simulink model "TosAeb"  (R2021a)
settings: Solver=FixedStepDiscrete  StartTime=0.0  StopTime=inf  FixedStep=0.01  RelTol=1e-3
undefined symbols (not defined in the file or in its folder): ACCModSts, AccSts1, AEB_ObjectMovingOrientation, AEB_ObjectMovingState, CN_BTCActive, CN_CurrentGearR, CN_CurrentGearR_DHT, CN_PTCActive, CN_VDCActive, CN_VSC_EspOff, CN_VSC_FCW_AEB_Error, CN_VSC_FCW_AEB_NoDispay, CN_VSC_FCW_AEB_PerfmDgdtn, CN_VSC_FCW_AEB_TempUnAvlbl, CN_VSC_VehStandstill, CNaccelAvoidMax, CNaccelAvoidMin, CNbrakePedalPress, CNcomfortStrLeftAccelMax, CNcomfortStrLeftAccelMin, CNcomfortStrRightAccelMax, CNcomfortStrRightAccelMin, CNDrvDoorOpen, CNDrvModeEco, CNDrvModeNorm, CNDrvModeOther, CNDrvModeSport, CNDrvSBRreminder, CNNull, CNobjInvalidID, CNPassDoorOpen, CNPassSBRreminder, CNPEBSAccelNegInf, CNPEBSAccelNull, CNPEBSTimeInf, CNPEBSTimeNull, CNSTOPMAX, CNSTOPNULL, CNsubjWidth, CNSwtOFF, CNSwtON, CNTTCMAX, CNTTCNULL, CNVehConfigAEBCycNone, CNVehConfigAEBNone, CNVehConfigAEBPedNone, CNVehConfigFCWNone, CNVehConfigJANone, CollisionSeverity, FALSE, FCWSnvty, JACycObjCalcInfoDefaultParams, JAPedObjCalcInfoDefaultParams, JAVehObjCalcInfoDefaultParams, latCycObjCalcInfoDefaultParams, latPedObjCalcInfoDefaultParams, longObjCalcInfoDefaultParams, ModSts, movingStatus, ObjectType ... (+1112)
libraries: simulink x3031, AEBSFunctionLib x50, Ta_Library x33, AEBSStateMachineLib x23, Mylibrary x12, simulink_extras x7, ObjSelect_lib x3, dspstat3 x2
(part 8 of 102)

== system TosAeb/FcwAebJa_Step_sys/ALG_AEBSystem/ALG_AEBController/AEB_FuncCondCalcApp/CycFunctionCondCalc ==
  inputSignals : Inport
  Bus Creator : BusCreator  Inputs=8  OutDataTypeStr=Bus: BusCycFuncSuppAborCond
      settings: InheritFromInputs=off
  cycAEBfullSuppAborCond : Subsystem
  cycAEBpartSuppAborCond : Subsystem
  cycPrefillSuppAborCond : Subsystem
  cycFuncSuppAborCond : Outport  OutDataTypeStr=Bus: BusCycFuncSuppAborCond
  wires:
  cycAEBfullSuppAborCond/1(cycAEBfullSuppCond) -> Bus Creator/6  "cycAEBfullSuppCond"
  cycAEBpartSuppAborCond/3(cycAEBpartAborCondStandstill) -> Bus Creator/5  "cycAEBpartAborCondStandstill"
  cycAEBfullSuppAborCond/2(cycAEBfullAborCondNoStandstill) -> Bus Creator/7  "cycAEBfullAborCondNoStandstill"
  cycAEBfullSuppAborCond/3(cycAEBfullAborCondStandstill) -> Bus Creator/8  "cycAEBfullAborCondStandstill"
  cycAEBpartSuppAborCond/2(cycAEBpartAborCondNoStandstill) -> Bus Creator/4  "cycAEBpartAborCondNoStandstill"
  inputSignals/1 -> cycPrefillSuppAborCond/1(inputSignals), cycAEBpartSuppAborCond/1(inputSignals), cycAEBfullSuppAborCond/1(inputSignals)
  cycAEBpartSuppAborCond/1(cycAEBpartSuppCond) -> Bus Creator/3  "cycAEBpartSuppCond"
  cycPrefillSuppAborCond/2(cycPrefillAborCond) -> Bus Creator/2  "cycPrefillAborCond"
  cycPrefillSuppAborCond/1(cycPrefillSuppCond) -> Bus Creator/1  "cycPrefillSuppCond"
  Bus Creator/1 -> cycFuncSuppAborCond/1

== system TosAeb/FcwAebJa_Step_sys/ALG_AEBSystem/ALG_AEBController/AEB_FuncCondCalcApp/JaCycFunctionCondCalc ==
  inputSignals : Inport
  Bus Creator : BusCreator  Inputs=5
  JaCycAEBfullSuppAborCond : Subsystem
  JaCycPrefillSuppAborCond : Subsystem
  JaCycFuncSuppAborCond : Outport
  wires:
  JaCycAEBfullSuppAborCond/3(JaCycAEBfullAborCondStandstill) -> Bus Creator/5  "JACycAEBfullAborCondStandstill"
  inputSignals/1 -> JaCycPrefillSuppAborCond/1(inputSignals), JaCycAEBfullSuppAborCond/1(inputSignals)
  JaCycPrefillSuppAborCond/2(JaCycPrefillAborCond) -> Bus Creator/2  "JACycPrefillAborCond"
  JaCycPrefillSuppAborCond/1(JaCycPrefillSuppCond) -> Bus Creator/1  "JACycPrefillSuppCond"
  Bus Creator/1 -> JaCycFuncSuppAborCond/1
  JaCycAEBfullSuppAborCond/1(JaCycAEBfullSuppCond) -> Bus Creator/3  "JACycAEBfullSuppCond"
  JaCycAEBfullSuppAborCond/2(JaCycAEBfullAborCondNoStandstill) -> Bus Creator/4  "JACycAEBfullAborCondNoStandstill"

== system TosAeb/FcwAebJa_Step_sys/ALG_AEBSystem/ALG_AEBController/AEB_FuncCondCalcApp/JaPedFunctionCondCalc ==
  inputSignals : Inport
  AEBJAPedAEBfullSuppAborCond : Subsystem
  AEBJAPedPrefillSuppAborCond : Subsystem
  Bus Creator : BusCreator  Inputs=5
  AEBJAPedFuncSuppAborCond : Outport
  wires:
  AEBJAPedAEBfullSuppAborCond/2(AEBJAPedAEBfullAborCondNoStandstill) -> Bus Creator/4  "JAPedAEBfullAborCondNoStandstill"
  AEBJAPedAEBfullSuppAborCond/1(AEBJAPedAEBfullSuppCond) -> Bus Creator/3  "JAPedAEBfullSuppCond"
  Bus Creator/1 -> AEBJAPedFuncSuppAborCond/1
  AEBJAPedPrefillSuppAborCond/1(AEBJAPedPrefillSuppCond) -> Bus Creator/1  "JAPedPrefillSuppCond"
  AEBJAPedPrefillSuppAborCond/2(AEBJAPedPrefillAborCond) -> Bus Creator/2  "JAPedPrefillAborCond"
  inputSignals/1 -> AEBJAPedAEBfullSuppAborCond/1(inputSignals), AEBJAPedPrefillSuppAborCond/1(inputSignals)
  AEBJAPedAEBfullSuppAborCond/3(AEBJAPedAEBfullAborCondStandstill) -> Bus Creator/5  "JAPedAEBfullAborCondStandstill"

== system TosAeb/FcwAebJa_Step_sys/ALG_AEBSystem/ALG_AEBController/AEB_FuncCondCalcApp/JaVehFunctionCondCalc ==
  inputSignals : Inport
  AEBJAVehAEBfullSuppAborCond : Subsystem
  AEBJAVehPrefillSuppAborCond : Subsystem
  Bus Creator : BusCreator  Inputs=5
  AEBJAVehFuncSuppAborCond : Outport
  wires:
  AEBJAVehAEBfullSuppAborCond/3(AEBJAVehAEBfullAborCondStandstill) -> Bus Creator/5  "JAVehAEBfullAborCondStandstill"
  inputSignals/1 -> AEBJAVehPrefillSuppAborCond/1(inputSignals), AEBJAVehAEBfullSuppAborCond/1(inputSignals)
  AEBJAVehPrefillSuppAborCond/2(AEBJAVehPrefillAborCond) -> Bus Creator/2  "JAVehPrefillAborCond"
  AEBJAVehPrefillSuppAborCond/1(AEBJAVehPrefillSuppCond) -> Bus Creator/1  "JAVehPrefillSuppCond"
  Bus Creator/1 -> AEBJAVehFuncSuppAborCond/1
  AEBJAVehAEBfullSuppAborCond/1(AEBJAVehAEBfullSuppCond) -> Bus Creator/3  "JAVehAEBfullSuppCond"
  AEBJAVehAEBfullSuppAborCond/2(AEBJAVehAEBfullAborCondNoStandstill) -> Bus Creator/4  "JAVehAEBfullAborCondNoStandstill"

== system TosAeb/FcwAebJa_Step_sys/ALG_AEBSystem/ALG_AEBController/AEB_FuncCondCalcApp/LongFunctionCondCalc ==
  inputSignals : Inport
  Bus Creator1 : BusCreator  Inputs=19  OutDataTypeStr=Bus: BusVehFuncSuppAborCond
      settings: InheritFromInputs=off
  vehABASuppAborCondCalc : Subsystem
  vehAEBfullSuppAborCondCalc : Subsystem
  vehAEBpartSuppAborCondCalc : Subsystem
  vehAcuteWarningSuppAborCondFulfilled : Subsystem
  vehEBASuppAborCondCalc : Subsystem
  vehLatentWarningSuppAborCondFulfilled : Subsystem
  vehPreWarningSuppAborCondFulfilled : Subsystem
  vehPrefillSuppAborCondCalc : Subsystem
  vehFuncSuppAborCond : Outport  OutDataTypeStr=Bus: BusVehFuncSuppAborCond
  wires:
  Bus Creator1/1 -> vehFuncSuppAborCond/1  "vehFuncSuppAborCond"
  vehAEBpartSuppAborCondCalc/3(vehAEBpartAborCondStandstill) -> Bus Creator1/13  "vehAEBpartAborCondStandstill"
  vehAEBpartSuppAborCondCalc/2(vehAEBpartAborCondNoStandstill) -> Bus Creator1/12  "vehAEBpartAborCondNoStandstill"
  vehPrefillSuppAborCondCalc/2(vehPrefillAborCond) -> Bus Creator1/8  "vehPrefillAborCond"
  vehAEBpartSuppAborCondCalc/1(vehAEBpartSuppCond) -> Bus Creator1/11  "vehAEBpartSuppCond"
  vehABASuppAborCondCalc/1(vehABASuppCond) -> Bus Creator1/9  "vehABASuppCond"
  vehAEBfullSuppAborCondCalc/2(vehAEBfullAborCondNoStandstill) -> Bus Creator1/15  "vehAEBfullAborCondNoStandstill"
  vehEBASuppAborCondCalc/1(vehEBASuppCond) -> Bus Creator1/17  "vehEBASuppCond"
  vehAEBfullSuppAborCondCalc/3(vehAEBfullAborCondStandstill) -> Bus Creator1/16  "vehAEBfullAborCondStandstill"
  vehPrefillSuppAborCondCalc/1(vehPrefillSuppCond) -> Bus Creator1/7  "vehPrefillSuppAborCond"
  vehAEBfullSuppAborCondCalc/1(vehAEBfullSuppCond) -> Bus Creator1/14  "vehAEBfullSuppCond"
  vehABASuppAborCondCalc/2(vehABAAborCond) -> Bus Creator1/10  "vehABAAborCond"
  vehEBASuppAborCondCalc/2(vehEBAAborCondNoStandstill) -> Bus Creator1/18  "vehEBAAborCondNoStandstill"
  vehEBASuppAborCondCalc/3(vehEBAAborCondStandstill) -> Bus Creator1/19  "vehEBAAborCondStandstill"
  vehLatentWarningSuppAborCondFulfilled/2(vehLatentWarningAborCond) -> Bus Creator1/6  "vehLatentWarningAborCond"
  vehLatentWarningSuppAborCondFulfilled/1(vehLatentWarningSuppCond) -> Bus Creator1/5  "vehLatentWarningSuppCond"
  vehAcuteWarningSuppAborCondFulfilled/2(vehAcuteWarningAborCond) -> Bus Creator1/4  "vehAcuteWarningAborCond"
  vehAcuteWarningSuppAborCondFulfilled/1(vehAcuteWarningSuppCond) -> Bus Creator1/3  "vehAcuteWarningSuppCond"
  vehPreWarningSuppAborCondFulfilled/2(vehPreWarningAborCond) -> Bus Creator1/2  "vehPreWarningAborCond"
  vehPreWarningSuppAborCondFulfilled/1(vehPreWarningSuppCond) -> Bus Creator1/1  "vehPreWarningSuppCond"
  inputSignals/1 -> vehPreWarningSuppAborCondFulfilled/1(inputSignals), vehAcuteWarningSuppAborCondFulfilled/1(inputSignals), vehLatentWarningSuppAborCondFulfilled/1(inputSignals), vehPrefillSuppAborCondCalc/1(inputSignals), vehABASuppAborCondCalc/1(inputSignals), vehAEBpartSuppAborCondCalc/1(inputSignals), vehAEBfullSuppAborCondCalc/1(inputSignals), vehEBASuppAborCondCalc/1(inputSignals)

== system TosAeb/FcwAebJa_Step_sys/ALG_AEBSystem/ALG_AEBController/AEB_FuncCondCalcApp/PedFunctionCondCalc ==
  inputSignals : Inport
  Bus Creator : BusCreator  Inputs=9  OutDataTypeStr=Bus: BusPedFuncSuppAborCond
      settings: InheritFromInputs=off
  pedABASuppAborCond : Subsystem
  pedAEBfullSuppAborCond : Subsystem
  pedPreWarningSuppAborCond : Subsystem
  pedPrefillSuppAborCond : Subsystem
  pedFuncSuppAborCond : Outport  OutDataTypeStr=Bus: BusPedFuncSuppAborCond
  wires:
  pedAEBfullSuppAborCond/3(pedAEBfullAborCondStandstill) -> Bus Creator/9  "pedAEBfullAborCondStandstill"
  pedAEBfullSuppAborCond/2(pedAEBfullAborCondNoStandstill) -> Bus Creator/8  "pedAEBfullAborCondNoStandstill"
  pedAEBfullSuppAborCond/1(pedAEBfullSuppCond) -> Bus Creator/7  "pedAEBfullSuppCond"
  pedABASuppAborCond/2(pedABAAborCond) -> Bus Creator/6  "pedABAAborCond"
  pedABASuppAborCond/1(pedABASuppCond) -> Bus Creator/5  "pedABASuppCond"
  Bus Creator/1 -> pedFuncSuppAborCond/1
  pedPrefillSuppAborCond/1(pedPrefillSuppCond) -> Bus Creator/1  "pedPrefillSuppCond"
  pedPrefillSuppAborCond/2(pedPrefillAborCond) -> Bus Creator/2  "pedPrefillAborCond"
  pedPreWarningSuppAborCond/2(pedPreWarningAborCond) -> Bus Creator/4  "pedPreWarningAborCond"
  pedPreWarningSuppAborCond/1(pedPreWarningSuppCond) -> Bus Creator/3  "pedPreWarningSuppCond"
  inputSignals/1 -> pedAEBfullSuppAborCond/1(inputSignals), pedABASuppAborCond/1(inputSignals), pedPreWarningSuppAborCond/1(inputSignals), pedPrefillSuppAborCond/1(inputSignals)

== system TosAeb/FcwAebJa_Step_sys/ALG_AEBSystem/ALG_AEBController/AEB_FuncCondCalcInfo_FUS/CycFunctionCondCalc ==
  inputSignals : Inport
  Bus Creator : BusCreator  Inputs=8
  cycAEBfullSuppAborCond : Subsystem
  cycAEBpartSuppAborCond : Subsystem
  cycPrefillSuppAborCond : Subsystem
  cycFuncSuppAborCond : Outport
  wires:
  cycAEBfullSuppAborCond/1(cycAEBfullSuppCond) -> Bus Creator/6  "cycAEBfullSuppCond"
  cycAEBpartSuppAborCond/3(cycAEBpartAborCondStandstill) -> Bus Creator/5  "cycAEBpartAborCondStandstill"
  cycAEBfullSuppAborCond/2(cycAEBfullAborCondNoStandstill) -> Bus Creator/7  "cycAEBfullAborCondNoStandstill"
  cycAEBfullSuppAborCond/3(cycAEBfullAborCondStandstill) -> Bus Creator/8  "cycAEBfullAborCondStandstill"
  cycAEBpartSuppAborCond/2(cycAEBpartAborCondNoStandstill) -> Bus Creator/4  "cycAEBpartAborCondNoStandstill"
  inputSignals/1 -> cycPrefillSuppAborCond/1(inputSignals), cycAEBpartSuppAborCond/1(inputSignals), cycAEBfullSuppAborCond/1(inputSignals)
  cycAEBpartSuppAborCond/1(cycAEBpartSuppCond) -> Bus Creator/3  "cycAEBpartSuppCond"
  cycPrefillSuppAborCond/2(cycPrefillAborCond) -> Bus Creator/2  "cycPrefillAborCond"
  cycPrefillSuppAborCond/1(cycPrefillSuppCond) -> Bus Creator/1  "cycPrefillSuppCond"
  Bus Creator/1 -> cycFuncSuppAborCond/1

== system TosAeb/FcwAebJa_Step_sys/ALG_AEBSystem/ALG_AEBController/AEB_FuncCondCalcInfo_FUS/JaCycFunctionCondCalc ==
  inputSignals : Inport
  Bus Creator : BusCreator  Inputs=5
  JaCycAEBfullSuppAborCond : Subsystem
  JaCycPrefillSuppAborCond : Subsystem
  JaCycFuncSuppAborCond : Outport
  wires:
  JaCycAEBfullSuppAborCond/3(JaCycAEBfullAborCondStandstill) -> Bus Creator/5  "JACycAEBfullAborCondStandstill"
  inputSignals/1 -> JaCycPrefillSuppAborCond/1(inputSignals), JaCycAEBfullSuppAborCond/1(inputSignals)
  JaCycPrefillSuppAborCond/2(JaCycPrefillAborCond) -> Bus Creator/2  "JACycPrefillAborCond"
  JaCycPrefillSuppAborCond/1(JaCycPrefillSuppCond) -> Bus Creator/1  "JACycPrefillSuppCond"
  Bus Creator/1 -> JaCycFuncSuppAborCond/1
  JaCycAEBfullSuppAborCond/1(JaCycAEBfullSuppCond) -> Bus Creator/3  "JACycAEBfullSuppCond"
  JaCycAEBfullSuppAborCond/2(JaCycAEBfullAborCondNoStandstill) -> Bus Creator/4  "JACycAEBfullAborCondNoStandstill"

== system TosAeb/FcwAebJa_Step_sys/ALG_AEBSystem/ALG_AEBController/AEB_FuncCondCalcInfo_FUS/JaPedFunctionCondCalc ==
  inputSignals : Inport
  AEBJAPedAEBfullSuppAborCond : Subsystem
  AEBJAPedPrefillSuppAborCond : Subsystem
  Bus Creator : BusCreator  Inputs=5
  AEBJAPedFuncSuppAborCond : Outport
  wires:
  AEBJAPedAEBfullSuppAborCond/2(AEBJAPedAEBfullAborCondNoStandstill) -> Bus Creator/4  "JAPedAEBfullAborCondNoStandstill"
  AEBJAPedAEBfullSuppAborCond/1(AEBJAPedAEBfullSuppCond) -> Bus Creator/3  "JAPedAEBfullSuppCond"
  Bus Creator/1 -> AEBJAPedFuncSuppAborCond/1
  AEBJAPedPrefillSuppAborCond/1(JaPedPrefillSuppCond) -> Bus Creator/1  "JAPedPrefillSuppCond"
  AEBJAPedPrefillSuppAborCond/2(JaPedPrefillAborCond) -> Bus Creator/2  "JAPedPrefillAborCond"
  inputSignals/1 -> AEBJAPedAEBfullSuppAborCond/1(inputSignals), AEBJAPedPrefillSuppAborCond/1(inputSignals)
  AEBJAPedAEBfullSuppAborCond/3(AEBJAPedAEBfullAborCondStandstill) -> Bus Creator/5  "JAPedAEBfullAborCondStandstill"

== system TosAeb/FcwAebJa_Step_sys/ALG_AEBSystem/ALG_AEBController/AEB_FuncCondCalcInfo_FUS/JaVehFunctionCondCalc ==
  inputSignals : Inport
  Bus Creator : BusCreator  Inputs=5
  JaVehAEBfullSuppAborCond : Subsystem
  JaVehPrefillSuppAborCond : Subsystem
  AEBJAVehFuncSuppAborCond : Outport
  wires:
  JaVehAEBfullSuppAborCond/3(JaVehAEBfullAborCondStandstill) -> Bus Creator/5  "JAVehAEBfullAborCondStandstill"
  inputSignals/1 -> JaVehPrefillSuppAborCond/1(inputSignals), JaVehAEBfullSuppAborCond/1(inputSignals)
  JaVehPrefillSuppAborCond/2(JaVehPrefillAborCond) -> Bus Creator/2  "JAVehPrefillAborCond"
  JaVehPrefillSuppAborCond/1(JaVehPrefillSuppCond) -> Bus Creator/1  "JAVehPrefillSuppCond"
  Bus Creator/1 -> AEBJAVehFuncSuppAborCond/1
  JaVehAEBfullSuppAborCond/1(JaVehAEBfullSuppCond) -> Bus Creator/3  "JAVehAEBfullSuppCond"
  JaVehAEBfullSuppAborCond/2(JaVehAEBfullAborCondNoStandstill) -> Bus Creator/4  "JAVehAEBfullAborCondNoStandstill"

== system TosAeb/FcwAebJa_Step_sys/ALG_AEBSystem/ALG_AEBController/AEB_FuncCondCalcInfo_FUS/LongFunctionCondCalc ==
  inputSignals : Inport
  Bus Creator1 : BusCreator  Inputs=19  OutDataTypeStr=Bus: BusVehFuncSuppAborCond
      settings: InheritFromInputs=off
  vehABASuppAborCondCalc : Subsystem
  vehAEBfullSuppAborCondCalc : Subsystem
  vehAEBpartSuppAborCondCalc : Subsystem
  vehAcuteWarningSuppAborCondFulfilled : Subsystem
  vehEBASuppAborCondCalc : Subsystem
  vehLatentWarningSuppAborCondFulfilled : Subsystem
  vehPreWarningSuppAborCondFulfilled : Subsystem
  vehPrefillSuppAborCondCalc : Subsystem
  vehFuncSuppAborCond : Outport
  wires:
  Bus Creator1/1 -> vehFuncSuppAborCond/1  "vehFuncSuppAborCond"
  vehAEBpartSuppAborCondCalc/3(vehAEBpartAborCondStandstill) -> Bus Creator1/13  "vehAEBpartAborCondStandstill"
  vehAEBpartSuppAborCondCalc/2(vehAEBpartAborCondNoStandstill) -> Bus Creator1/12  "vehAEBpartAborCondNoStandstill"
  vehPrefillSuppAborCondCalc/2(vehPrefillAborCond) -> Bus Creator1/8  "vehPrefillAborCond"
  vehAEBpartSuppAborCondCalc/1(vehAEBpartSuppCond) -> Bus Creator1/11  "vehAEBpartSuppCond"
  vehABASuppAborCondCalc/1(vehABASuppCond) -> Bus Creator1/9  "vehABASuppCond"
  vehAEBfullSuppAborCondCalc/2(vehAEBfullAborCondNoStandstill) -> Bus Creator1/15  "vehAEBfullAborCondNoStandstill"
  vehEBASuppAborCondCalc/1(vehEBASuppCond) -> Bus Creator1/17  "vehEBASuppCond"
  vehAEBfullSuppAborCondCalc/3(vehAEBfullAborCondStandstill) -> Bus Creator1/16  "vehAEBfullAborCondStandstill"
  vehPrefillSuppAborCondCalc/1(vehPrefillSuppCond) -> Bus Creator1/7  "vehPrefillSuppAborCond"
  vehAEBfullSuppAborCondCalc/1(vehAEBfullSuppCond) -> Bus Creator1/14  "vehAEBfullSuppCond"
  vehABASuppAborCondCalc/2(vehABAAborCond) -> Bus Creator1/10  "vehABAAborCond"
  vehEBASuppAborCondCalc/2(vehEBAAborCondNoStandstill) -> Bus Creator1/18  "vehEBAAborCondNoStandstill"
  vehEBASuppAborCondCalc/3(vehEBAAborCondStandstill) -> Bus Creator1/19  "vehEBAAborCondStandstill"
  vehLatentWarningSuppAborCondFulfilled/2(vehLatentWarningAborCond) -> Bus Creator1/6  "vehLatentWarningAborCond"
  vehLatentWarningSuppAborCondFulfilled/1(vehLatentWarningSuppCond) -> Bus Creator1/5  "vehLatentWarningSuppCond"
  vehAcuteWarningSuppAborCondFulfilled/2(vehAcuteWarningAborCond) -> Bus Creator1/4  "vehAcuteWarningAborCond"
  vehAcuteWarningSuppAborCondFulfilled/1(vehAcuteWarningSuppCond) -> Bus Creator1/3  "vehAcuteWarningSuppCond"
  vehPreWarningSuppAborCondFulfilled/2(vehPreWarningAborCond) -> Bus Creator1/2  "vehPreWarningAborCond"
  vehPreWarningSuppAborCondFulfilled/1(vehPreWarningSuppCond) -> Bus Creator1/1  "vehPreWarningSuppCond"
  inputSignals/1 -> vehPreWarningSuppAborCondFulfilled/1(inputSignals), vehLatentWarningSuppAborCondFulfilled/1(inputSignals), vehPrefillSuppAborCondCalc/1(inputSignals), vehABASuppAborCondCalc/1(inputSignals), vehAEBpartSuppAborCondCalc/1(inputSignals), vehAEBfullSuppAborCondCalc/1(inputSignals), vehEBASuppAborCondCalc/1(inputSignals), vehAcuteWarningSuppAborCondFulfilled/1(inputSignals)

== system TosAeb/FcwAebJa_Step_sys/ALG_AEBSystem/ALG_AEBController/AEB_FuncCondCalcInfo_FUS/PedFunctionCondCalc ==
  inputSignals : Inport
  Bus Creator : BusCreator  Inputs=9
  pedABASuppAborCond : Subsystem
  pedAEBfullSuppAborCond : Subsystem
  pedPreWarningSuppAborCond : Subsystem
  pedPrefillSuppAborCond : Subsystem
  pedFuncSuppAborCond : Outport
  wires:
  pedAEBfullSuppAborCond/3(pedAEBfullAborCondStandstill) -> Bus Creator/9  "pedAEBfullAborCondStandstill"
  pedAEBfullSuppAborCond/2(pedAEBfullAborCondNoStandstill) -> Bus Creator/8  "pedAEBfullAborCondNoStandstill"
  pedAEBfullSuppAborCond/1(pedAEBfullSuppCond) -> Bus Creator/7  "pedAEBfullSuppCond"
  pedABASuppAborCond/2(pedABAAborCond) -> Bus Creator/6  "pedABAAborCond"
  pedABASuppAborCond/1(pedABASuppCond) -> Bus Creator/5  "pedABASuppCond"
  Bus Creator/1 -> pedFuncSuppAborCond/1
  pedPrefillSuppAborCond/1(pedPrefillSuppCond) -> Bus Creator/1  "pedPrefillSuppCond"
  pedPrefillSuppAborCond/2(pedPrefillAborCond) -> Bus Creator/2  "pedPrefillAborCond"
  pedPreWarningSuppAborCond/2(pedPreWarningAborCond) -> Bus Creator/4  "pedPreWarningAborCond"
  pedPreWarningSuppAborCond/1(pedPreWarningSuppCond) -> Bus Creator/3  "pedPreWarningSuppCond"
  inputSignals/1 -> pedAEBfullSuppAborCond/1(inputSignals), pedABASuppAborCond/1(inputSignals), pedPreWarningSuppAborCond/1(inputSignals), pedPrefillSuppAborCond/1(inputSignals)

== system TosAeb/FcwAebJa_Step_sys/ALG_AEBSystem/ALG_AEBController/AEB_FuncImpManagementApp/FuncBlockTimeCalc ==
  AEBModStsFuncImpArb : Inport  OutDataTypeStr=Bus: BusAEBModStsFuncImpArb
  Bus Creator : BusCreator  Inputs=3  OutDataTypeStr=Bus: BusFuncBlockTime  NonVirtualBus=on
      settings: InheritFromInputs=off
  tBlockTimeBrkCalc : Subsystem
  tBlockTimeBrkReadyCalc : Subsystem
  tBlockTimeWarningCalc : Subsystem
  funcBlockTime : Outport  OutDataTypeStr=Bus: BusFuncBlockTime
  wires:
  Bus Creator/1 -> funcBlockTime/1
  tBlockTimeBrkCalc/1(tBlockTimeBrk) -> Bus Creator/3  "tBlockTimeBrk"
  tBlockTimeWarningCalc/1(tBlockTimeWarning) -> Bus Creator/2  "tBlockTimeWarning"
  tBlockTimeBrkReadyCalc/1(tBlockTimeBrkReady) -> Bus Creator/1  "tBlockTimeBrkReady"
  AEBModStsFuncImpArb/1 -> tBlockTimeBrkReadyCalc/1(AEBModStsFuncImpArb), tBlockTimeWarningCalc/1(AEBModStsFuncImpArb), tBlockTimeBrkCalc/1(AEBModStsFuncImpArb)

== system TosAeb/FcwAebJa_Step_sys/ALG_AEBSystem/ALG_AEBController/AEB_FuncImpManagementApp/FuncImpArbCalc ==
  AEBModStsArb : Inport  OutDataTypeStr=Bus: BusAEBModStsArb
  FCTBModStsArb : Inport  Port=2  OutDataTypeStr=Enum: ModSts
  FCTB_ABAModStsArb : Inport  Port=3  OutDataTypeStr=Enum: ModSts
  FCTB_PrefillModStsArb : Inport  Port=4  OutDataTypeStr=Enum: ModSts
  BrkFuncImpArbCalc : Subsystem
  BrkFuncImpArbCalc1 : Subsystem  Commented=on
  BrkReadyFuncImpArbCalc : Subsystem
  BrkReadyFuncImpArbCalc1 : Subsystem  Commented=on
  Bus Creator : BusCreator  Inputs=3  OutDataTypeStr=Bus: BusAEBModStsFuncImpArb  NonVirtualBus=on
      settings: InheritFromInputs=off
  Bus Creator1 : BusCreator  Inputs=3  OutDataTypeStr=Bus: BusFuncImpSuppAborCond  NonVirtualBus=on
      settings: InheritFromInputs=off
  Bus Selector : BusSelector  OutputSignals=vehAEBModStsArb.prefillModStsArb,vehAEBModStsArb.ABAModStsArb,latAEBModStsArb.pedPrefillModStsArb,latAEBModStsArb.pedABAModStsArb,latAEBModStsArb.cycPrefillModStsArb,JA_AEBModStsArb.JA_vehPrefillModStsArb,JA_AEBModStsArb.JA_PTW_PrefillModStsArb,JA_AEBModStsArb.JA_cycPrefillModStsArb,JA_AEBModStsArb.JA_pedPrefillModStsArb
  Bus Selector1 : BusSelector  OutputSignals=vehAEBModStsArb.AEBpartModStsArb,vehAEBModStsArb.AEBfullModStsArb,vehAEBModStsArb.EBAModStsArb,latAEBModStsArb.cycAEBpartModStsArb,latAEBModStsArb.cycAEBfullModStsArb,latAEBModStsArb.pedAEBfullModStsArb,JA_AEBModStsArb.JA_vehAEBModStsArb,JA_AEBModStsArb.JA_PTW_AEBModStsArb,JA_AEBModStsArb.JA_cycAEBModStsArb,JA_AEBModStsArb.JA_pedAEBModStsArb,JA_AEBModStsArb.JA_pedAEBModStsArb,JA_AEBModStsArb.JA_cycAEBModStsArb,JA_AEBModStsArb.JA_PTW_AEBModStsArb
  Terminator : Terminator
  WarningFuncImpArbCalc : Subsystem
  AEBModStsFuncImpArb : Outport
  FuncImpSuppCondAbor : Outport  Port=2
  wires:
  FCTBModStsArb/1 -> BrkFuncImpArbCalc/14(FCTBModStsArb)
  Bus Selector1/13 -> BrkFuncImpArbCalc/13(JA_PTW_AEBfullModStsArb)  "<JA_PTW_AEBModStsArb>"
  Bus Selector1/12 -> BrkFuncImpArbCalc/12(JA_cycAEBfullModStsArb)  "<JA_cycAEBModStsArb>"
  Bus Selector1/11 -> BrkFuncImpArbCalc/11(JA_pedAEBfullModStsArb)  "<JA_pedAEBModStsArb>"
  Bus Selector1/10 -> BrkFuncImpArbCalc/10(JA_pedAEBModStsArb)  "<JA_pedAEBModStsArb>"
  Bus Selector1/9 -> BrkFuncImpArbCalc/9(JA_cycAEBModStsArb)  "<JA_cycAEBModStsArb>"
  Bus Selector1/8 -> BrkFuncImpArbCalc/8(JA_PTW_AEBModStsArb)  "<JA_PTW_AEBModStsArb>"
  Bus Selector1/7 -> BrkFuncImpArbCalc/7(JA_vehAEBModStsArb)  "<JA_vehAEBModStsArb>"
  Bus Selector1/6 -> BrkFuncImpArbCalc/6(pedAEBfullModStsArb)  "<pedAEBfullModStsArb>"
  Bus Selector1/5 -> BrkFuncImpArbCalc/5(cycAEBfullModStsArb)  "<cycAEBfullModStsArb>"
  Bus Selector1/4 -> BrkFuncImpArbCalc/4(cycAEBpartModStsArb)  "<cycAEBpartModStsArb>"
  Bus Selector1/3 -> BrkFuncImpArbCalc/3(EBAModStsArb)  "<EBAModStsArb>"
  Bus Selector1/2 -> BrkFuncImpArbCalc/2(AEBfullModStsArb)  "<AEBfullModStsArb>"
  Bus Selector1/1 -> BrkFuncImpArbCalc/1(AEBpartModStsArb)  "<AEBpartModStsArb>"
  FCTB_PrefillModStsArb/1 -> BrkReadyFuncImpArbCalc/10(FCTB_PrefillModStsArb)  "FCTB_PrefillModStsArb"
  FCTB_ABAModStsArb/1 -> BrkReadyFuncImpArbCalc/11(FCTB_ABAModStsArb)  "FCTB_ABAModStsArb"
  Bus Selector/9 -> BrkReadyFuncImpArbCalc/9(JA_pedPrefillModStsArb)  "<JA_pedPrefillModStsArb>"
  Bus Selector/8 -> BrkReadyFuncImpArbCalc/8(JA_cycPrefillModStsArb)  "<JA_cycPrefillModStsArb>"
  Bus Selector/7 -> BrkReadyFuncImpArbCalc/7(JA_PTW_PrefillModStsArb)  "<JA_PTW_PrefillModStsArb>"
  Bus Selector/6 -> BrkReadyFuncImpArbCalc/6(JA_vehPrefillModStsArb)  "<JA_vehPrefillModStsArb>"
  Bus Selector/5 -> BrkReadyFuncImpArbCalc/5(cycPrefillModStsArb)  "<cycPrefillModStsArb>"
  Bus Selector/4 -> BrkReadyFuncImpArbCalc/4(pedABAModStsArb)  "<pedABAModStsArb>"
  Bus Selector/3 -> BrkReadyFuncImpArbCalc/3(pedPrefillModStsArb)  "<pedPrefillModStsArb>"
  Bus Selector/2 -> BrkReadyFuncImpArbCalc/2(ABAModStsArb)  "<ABAModStsArb>"
  Bus Selector/1 -> BrkReadyFuncImpArbCalc/1(prefillModStsArb)  "<prefillModStsArb>"
  BrkFuncImpArbCalc/2(brkFuncImpArb) -> Bus Creator/3  "brkFuncImpArb"
  WarningFuncImpArbCalc/3(warningFuncImpArb) -> Bus Creator/1  "warningFuncImpArb"
  Bus Creator/1 -> AEBModStsFuncImpArb/1
  BrkFuncImpArbCalc/3(brkFuncImpSuppCondAbor) -> Bus Creator1/3  "brkFuncImpSuppAborCond"
  BrkReadyFuncImpArbCalc/2(brkReadyFuncImpArb) -> Bus Creator/2  "brkReadyFuncImpArb"
  WarningFuncImpArbCalc/1(warningFuncActvCond) -> Terminator/1
  [warningFuncImpSuppAbor] -> Bus Creator1/1  "warningFuncImpSuppAborCond"
  BrkReadyFuncImpArbCalc/3(brkReadyFuncImpSuppCondAbor) -> [brkReadyFuncImpSuppAbor]
  Bus Creator1/1 -> FuncImpSuppCondAbor/1
  [brkReadyFuncImpSuppAbor] -> Bus Creator1/2  "brkReadyFuncImpSuppAborCond"
  WarningFuncImpArbCalc/2(warningFuncImpSuppCondAbor) -> [warningFuncImpSuppAbor]
  BrkFuncImpArbCalc/1(brkFuncActvCond) -> [brkFuncActvCond], BrkReadyFuncImpArbCalc/12(brkFuncActiveCond)
  AEBModStsArb/1 -> Bus Selector1/1, Bus Selector/1, WarningFuncImpArbCalc/1(AEBModStsArb)
  [brkFuncActvCond] -> WarningFuncImpArbCalc/3(brkFuncActvCond)
  BrkReadyFuncImpArbCalc/1(brkReadyFuncActvCond) -> WarningFuncImpArbCalc/2(brkReadyFuncActvCond)

== system TosAeb/FcwAebJa_Step_sys/ALG_AEBSystem/ALG_AEBController/AEB_FuncImpManagementApp/FuncManeuArbCalc ==
  AEBModStsFuncImpArb : Inport  OutDataTypeStr=Bus: BusAEBModStsFuncImpArb
  AEBAvailable : Inport  Port=2
  ObjectMovingState : Inport  Port=3
  accelAvoid : Inport  Port=4
  axAbsObj : Inport  Port=5
  AEBBA : Inport  Port=6
  AEBIB : Inport  Port=7
  VehicleStandstill : Inport  Port=8
  VSC_VehStandstill : Inport  Port=9  OutDataTypeStr=boolean
  ABPAvailable : Inport  Port=10
  ABAAvailable : Inport  Port=11
  longCollisionSeverity : Inport  Port=12
  ABPActive : Inport  Port=13
  ABAActive : Inport  Port=14
  FCTB_ABA_Level : Inport  Port=15
  subjVel : Inport  Port=16
  AWBActive : Inport  Port=17
  vx : Inport  Port=18
  longObjCalcInfo : Inport  Port=19  OutDataTypeStr=Bus: BusLongObjCalcInfo
  latPedTgtCalcInfo : Inport  Port=20  OutDataTypeStr=Bus: BusLatPedTgtCalcInfo
  FCTB_BrkgReqVal : Inport  Port=21
  subjLongAccel : Inport  Port=22
  BrkReadyManeuArbCalc : Subsystem
  Bus Selector : BusSelector  OutputSignals=brkFuncImpArb
  Bus Selector1 : BusSelector  OutputSignals=brkReadyFuncImpArb
  Bus Selector2 : BusSelector  OutputSignals=latPedCollsionAssesInfo.latCollisionSeverity
  Terminator : Terminator
  WarningManeuArbCalc : Subsystem
  brkFuncManeuArbCalc : Subsystem
  Bus Element Out : Outport  PortName=AEBSFuncOutput  Element=AEBTrigger
  Out Bus Element : Outport  PortName=AEBSFuncOutput  Element=axTgtReqValue
  Out Bus Element1 : Outport  PortName=AEBSFuncOutput  Element=ABPTrigger
  Out Bus Element2 : Outport  PortName=AEBSFuncOutput  Element=ABATrigger
  Out Bus Element3 : Outport  PortName=AEBSFuncOutput  Element=ABALvl
  Out Bus Element4 : Outport  PortName=AEBSFuncOutput  Element=latentWarningTrigger
  Out Bus Element5 : Outport  PortName=AEBSFuncOutput  Element=preWarningTrigger
  Out Bus Element6 : Outport  PortName=AEBSFuncOutput  Element=acuteWarningTrigger
  Out Bus Element7 : Outport  PortName=AEBSFuncOutput  Element=acuteWarningLvl
  Bus Element Out1 : Outport  Port=2  PortName=funcManeuAborCond  Element=vehBrkManeuAborCond
  Out Bus Element8 : Outport  Port=2  PortName=funcManeuAborCond  Element=latBrkManeuAborCond
  Out Bus Element9 : Outport  Port=2  PortName=funcManeuAborCond  Element=JABrkManeuAborCond
  Out Bus Element10 : Outport  Port=2  PortName=funcManeuAborCond  Element=ABPFuncManeuAborCond
  Out Bus Element11 : Outport  Port=2  PortName=funcManeuAborCond  Element=ABAFuncManeuAborCond
  Out Bus Element12 : Outport  Port=2  PortName=funcManeuAborCond  Element=acuteWarningFuncManeuAborCond
  wires:
  subjLongAccel/1 -> brkFuncManeuArbCalc/14(subjLongAccel)
  FCTB_BrkgReqVal/1 -> brkFuncManeuArbCalc/13(FCTB_BrkgReqVal)
  VehicleStandstill/1 -> brkFuncManeuArbCalc/11(VehicleStandstill)
  FCTB_ABA_Level/1 -> BrkReadyManeuArbCalc/8(FCTB_ABA_Level)
  latPedTgtCalcInfo/1 -> Bus Selector2/1
  longObjCalcInfo/1 -> brkFuncManeuArbCalc/10(longObjCalcInfo)
  vx/1 -> brkFuncManeuArbCalc/9(vx)
  Bus Selector1/1 -> BrkReadyManeuArbCalc/1(breakReadyFuncImpArb)  "<brkReadyFuncImpArb>"
  Bus Selector/1 -> brkFuncManeuArbCalc/1(brkFuncImpArb)  "<brkFuncImpArb>"
  longCollisionSeverity/1 -> BrkReadyManeuArbCalc/4(longCollisionSeverity)
  [subjVel] -> brkFuncManeuArbCalc/8(subjVel)
  brkFuncManeuArbCalc/3(axTgtReqValueManeu) -> Out Bus Element/1
  brkFuncManeuArbCalc/6(JABrkManeuAbor) -> Out Bus Element9/1
  brkFuncManeuArbCalc/5(latBrkManeuAbor) -> Out Bus Element8/1
  brkFuncManeuArbCalc/4(vehBrkManeuAbor) -> Bus Element Out1/1
  brkFuncManeuArbCalc/1(AEBTrigger) -> Terminator/1
  AWBActive/1 -> WarningManeuArbCalc/3(AWBActive)
  subjVel/1 -> WarningManeuArbCalc/2(subjVel), [subjVel]
  ABPActive/1 -> BrkReadyManeuArbCalc/6(ABPActive)
  Bus Selector2/1 -> BrkReadyManeuArbCalc/5(pedCollisionSeverity)  "<latCollisionSeverity>"
  ABAAvailable/1 -> BrkReadyManeuArbCalc/3(ABAAvailable)
  ABPAvailable/1 -> BrkReadyManeuArbCalc/2(ABPAvailable)
  ABAActive/1 -> BrkReadyManeuArbCalc/7(ABAActive)
  axAbsObj/1 -> brkFuncManeuArbCalc/5(axAbsObj)
  accelAvoid/1 -> brkFuncManeuArbCalc/4(accelAvoid)
  ObjectMovingState/1 -> brkFuncManeuArbCalc/3(ObjectMovingState)
  AEBIB/1 -> brkFuncManeuArbCalc/7(AEBIB)
  AEBBA/1 -> brkFuncManeuArbCalc/6(AEBBA)
  AEBModStsFuncImpArb/1 -> WarningManeuArbCalc/1(AEBModStsFuncImpArb), Bus Selector1/1, Bus Selector/1
  AEBAvailable/1 -> brkFuncManeuArbCalc/2(AEBAvailable)
  brkFuncManeuArbCalc/2(AEBTriggerManeu) -> Bus Element Out/1
  WarningManeuArbCalc/5(acuteWarningFuncManeuAbor) -> Out Bus Element12/1
  WarningManeuArbCalc/4(acuteWarningLvl) -> Out Bus Element7/1
  WarningManeuArbCalc/3(acuteWarningTrigger) -> Out Bus Element6/1
  WarningManeuArbCalc/2(preWarningTrigger) -> Out Bus Element5/1
  WarningManeuArbCalc/1(latentWarningTrigger) -> Out Bus Element4/1
  BrkReadyManeuArbCalc/5(ABAFuncManeuAbor) -> Out Bus Element11/1
  BrkReadyManeuArbCalc/4(ABPFuncManeuAbor) -> Out Bus Element10/1
  BrkReadyManeuArbCalc/3(ABALvl) -> Out Bus Element3/1
  BrkReadyManeuArbCalc/2(ABATrigger) -> Out Bus Element2/1
  BrkReadyManeuArbCalc/1(ABPTrigger) -> Out Bus Element1/1
  VSC_VehStandstill/1 -> brkFuncManeuArbCalc/12(VSC_VehStandstill)  "VSC_VehStandstill"

== system TosAeb/FcwAebJa_Step_sys/ALG_AEBSystem/ALG_AEBController/AEB_JaObjCalcApp/JAPedTgtInfoCalc ==
  note: AEBS Junction Assist VRU Object Information Calculation
  AEBJAObj : Inport  OutDataTypeStr=Bus: BusAEBJAObjBus  PortDimensions=32  BusOutputAsStruct=on
  subjInfo : Inport  Port=2  OutDataTypeStr=Bus: BusSubjInfo
  SteerWheelAngle_Deg : Inport  Port=3
  JAPedPrefillModSts : Inport  Port=4
  JAPedAEBfullModSts : Inport  Port=5
  Bus Creator : BusCreator  Inputs=2  OutDataTypeStr=Bus: BusAEBJAPedTgtCalcInfo  NonVirtualBus=on
      settings: InheritFromInputs=off
  Bus Selector : BusSelector  OutputSignals=subjVel
  Bus Selector1 : BusSelector  OutputSignals=AEBJAPedObj.ObjectID,AEBJAPedObj.vx,AEBJAPedObj.vy,AEBJAPedObj.dx,AEBJAPedObj.dy,AEBJAPedObjAbsInfo.dxFrontBumper,AEBJAPedObjColliInfo.tPredictTime
  Bus Selector2 : BusSelector  OutputSignals=subjYawRate,subjVel,subjLongAccel
  JAPedCollisonAssessInfo : Subsystem
  JAPedObjCalcInfoCalc : Subsystem  TreatAsAtomicUnit=on
  TargetSelectCalc : Subsystem
  AEBJAPedTgtCalcInfo : Outport  OutDataTypeStr=Bus: BusAEBJAPedTgtCalcInfo
  wires:
  subjInfo/1 -> JAPedObjCalcInfoCalc/2(BusSubjInfo), Bus Selector2/1, Bus Selector/1
  TargetSelectCalc/1(JAPedTgtObjInfo) -> Bus Selector1/1, Bus Creator/1  "JAPedTgtObjInfo"
  Bus Selector/1 -> TargetSelectCalc/3(subjVel)  "<subjVel>"
  JAPedPrefillModSts/1 -> TargetSelectCalc/5(JAPedPrefillModSts)
  Bus Selector1/3 -> JAPedCollisonAssessInfo/3(vy)  "<vy>"
  Bus Selector1/1 -> JAPedCollisonAssessInfo/1(ObjectID)  "<ObjectID>"
  Bus Selector1/2 -> JAPedCollisonAssessInfo/2(vx)  "<vx>"
  JAPedAEBfullModSts/1 -> TargetSelectCalc/6(JAPedAEBfullModSts)
  AEBJAObj/1 -> JAPedObjCalcInfoCalc/1(BusAEBJaObj)
  Bus Selector1/4 -> JAPedCollisonAssessInfo/4(dx)  "<dx>"
  JAPedCollisonAssessInfo/1(JAPedCollisonAssessInfo) -> Bus Creator/2  "JAPedCollisonAssessInfo"
  Bus Selector2/3 -> JAPedCollisonAssessInfo/10(subjLongAccel)  "<subjLongAccel>"
  Bus Selector2/1 -> JAPedCollisonAssessInfo/8(subjYawRate)  "<subjYawRate>"
  Bus Selector1/6 -> JAPedCollisonAssessInfo/6(dxFrontBumper)  "<dxFrontBumper>"
  Bus Selector2/2 -> JAPedCollisonAssessInfo/9(subjVel)  "<subjVel>"
  Bus Selector1/5 -> JAPedCollisonAssessInfo/5(dy)  "<dy>"
  Bus Creator/1 -> AEBJAPedTgtCalcInfo/1
  SteerWheelAngle_Deg/1 -> TargetSelectCalc/4(SteerWheelAngle_Deg)
  Bus Selector1/7 -> JAPedCollisonAssessInfo/7(tPredictTime)  "<tPredictTime>"
  JAPedObjCalcInfoCalc/1(JAPedObjCalcInfo) -> TargetSelectCalc/1(JAPedObjCalcInfo)  "AEB_JaPedObjCalcInfo"
  JAPedObjCalcInfoCalc/2(ObjPreSelectCond) -> TargetSelectCalc/2(ObjPreSelectCond)  "AEB_JaPedObjPreSelectCond"

== system TosAeb/FcwAebJa_Step_sys/ALG_AEBSystem/ALG_AEBController/AEB_JaObjCalcApp/JAVehTgtInfoCalc ==
  note: AEBS Junction Assist Vehicle Object Information Calculation
  AEBJAObj : Inport  OutDataTypeStr=Bus: BusAEBJAObjBus  PortDimensions=32  BusOutputAsStruct=on
  subjInfo : Inport  Port=2  OutDataTypeStr=Bus: BusSubjInfo
  SteerWheelAngle_Deg : Inport  Port=3  OutDataTypeStr=single
  JAVehPrefillModSts : Inport  Port=4
  JAVehAEBfullModSts : Inport  Port=5
  AEBJAVehObjCalc : Subsystem  TreatAsAtomicUnit=on
  Bus Creator : BusCreator  Inputs=2  OutDataTypeStr=Bus: BusAEBJAVehTgtCalcInfo  NonVirtualBus=on
      settings: InheritFromInputs=off
  Bus Selector : BusSelector  OutputSignals=AEBJAVehObj.ObjectID,AEBJAVehObj.vx,AEBJAVehObj.vy,AEBJAVehObj.dx,AEBJAVehObj.dy,AEBJAVehObjAbsInfo.dxFrontBumper,AEBJAVehObjColliInfo.tPredictTime
  Bus Selector1 : BusSelector  OutputSignals=subjYawRate,subjVel,subjLongAccel
  Bus Selector2 : BusSelector  OutputSignals=subjVel
  JAVehCollisonAssessInfoCalc : Subsystem
  TargetFinalSelect : Subsystem
  JAVehTgtCalcInfo : Outport  OutDataTypeStr=Bus: BusAEBJAVehTgtCalcInfo
  wires:
  Bus Selector/7 -> JAVehCollisonAssessInfoCalc/7(tPredictTime)  "<tPredictTime>"
  Bus Selector1/1 -> JAVehCollisonAssessInfoCalc/8(subjYawRate)  "<subjYawRate>"
  Bus Selector2/1 -> TargetFinalSelect/3(subjVel)  "<subjVel>"
  JAVehAEBfullModSts/1 -> TargetFinalSelect/6(JAVehAEBfullModSts)
  subjInfo/1 -> Bus Selector1/1, Bus Selector2/1, AEBJAVehObjCalc/2(subjInfo)
  JAVehPrefillModSts/1 -> TargetFinalSelect/5(JAVehPrefillModSts)
  Bus Selector/2 -> JAVehCollisonAssessInfoCalc/2(vx)  "<vx>"
  Bus Selector/4 -> JAVehCollisonAssessInfoCalc/4(dx)  "<dx>"
  Bus Selector/6 -> JAVehCollisonAssessInfoCalc/6(dxFrontBumper)  "<dxFrontBumper>"
  TargetFinalSelect/1(JAVehTgtObjInfo) -> Bus Selector/1, Bus Creator/1  "JAVehTgtObjInfo"
  AEBJAObj/1 -> AEBJAVehObjCalc/1(AEBJaVehObj)
  Bus Selector1/3 -> JAVehCollisonAssessInfoCalc/10(subjLongAccel)  "<subjLongAccel>"
  Bus Selector1/2 -> JAVehCollisonAssessInfoCalc/9(subjVel)  "<subjVel>"
  Bus Creator/1 -> JAVehTgtCalcInfo/1
  Bus Selector/1 -> JAVehCollisonAssessInfoCalc/1(ObjectID)  "<ObjectID>"
  Bus Selector/5 -> JAVehCollisonAssessInfoCalc/5(dy)  "<dy>"
  JAVehCollisonAssessInfoCalc/1(JAVehCollisonAssessInfo) -> Bus Creator/2  "JAVehCollisonAssessInfo"
  Bus Selector/3 -> JAVehCollisonAssessInfoCalc/3(vy)  "<vy>"
  SteerWheelAngle_Deg/1 -> TargetFinalSelect/4(SteerWheelAngle_Deg)
  AEBJAVehObjCalc/1(JaVehObjCalcInfo) -> TargetFinalSelect/1(JAVehObjCalcInfo)  "AEB_JaVehObjCalcInfo"
  AEBJAVehObjCalc/2(ObjPreSelectCond) -> TargetFinalSelect/2(ObjPreSelectCond)  "AEB_JaVehObjPreSelectCond"

== system TosAeb/FcwAebJa_Step_sys/ALG_AEBSystem/ALG_AEBController/AEB_JaObjCalcApp/JaCycTgtInfoCalc ==
  JaObj : Inport  OutDataTypeStr=Bus: BusAEBJAObjBus  PortDimensions=32  BusOutputAsStruct=on
  subjInfo : Inport  Port=2  OutDataTypeStr=Bus: BusSubjInfo
  SteerWheelAngle_Deg : Inport  Port=3
  JaCycPrefillModSts : Inport  Port=4
  JaCycAEBfullModSts : Inport  Port=5
  Bus Creator : BusCreator  Inputs=2  OutDataTypeStr=Bus: BusAEBJACycTgtCalcInfo  NonVirtualBus=on
      settings: InheritFromInputs=off
  Bus Selector : BusSelector  OutputSignals=subjVel
  Bus Selector1 : BusSelector  OutputSignals=JaCycObj.ObjectID,JaCycObj.vx,JaCycObj.vy,JaCycObj.dx,JaCycObj.dy,JaCycObjAbsInfo.dxFrontBumper,JaCycObjColliInfo.tPredictTime
  Bus Selector2 : BusSelector  OutputSignals=subjYawRate,subjVel,subjLongAccel
  JaCycCollisonAssessInfo : Subsystem
  JaCycObjCalcInfoCalc : Subsystem  TreatAsAtomicUnit=on
  TargetSelectCalc : Subsystem
  JaCycTgtCalcInfo : Outport  OutDataTypeStr=Bus: BusAEBJACycTgtCalcInfo
  wires:
  JaCycObjCalcInfoCalc/1(JaCycObjCalcInfo) -> TargetSelectCalc/1(JaCycObjCalcInfo)  "AEB_JaCycObjCalcInfo"
  Bus Selector1/7 -> JaCycCollisonAssessInfo/7(tPredictTime)  "<tPredictTime>"
  JaObj/1 -> JaCycObjCalcInfoCalc/1(JaObj)
  JaCycObjCalcInfoCalc/2(ObjPreSelectCond) -> TargetSelectCalc/2(ObjPreSelectCond)  "AEB_JaCycObjPreSelectCond"
  Bus Selector1/2 -> JaCycCollisonAssessInfo/2(vx)  "<vx>"
  Bus Selector1/1 -> JaCycCollisonAssessInfo/1(ObjectID)  "<ObjectID>"
  JaCycAEBfullModSts/1 -> TargetSelectCalc/6(JaCycAEBfullModSts)
  Bus Selector2/3 -> JaCycCollisonAssessInfo/10(subjLongAccel)  "<subjLongAccel>"
  Bus Selector1/6 -> JaCycCollisonAssessInfo/6(dxFrontBumper)  "<dxFrontBumper>"
  TargetSelectCalc/1(JaCycTgtObjInfo) -> Bus Selector1/1, Bus Creator/1  "JACycTgtObjInfo"
  Bus Selector1/5 -> JaCycCollisonAssessInfo/5(dy)  "<dy>"
  Bus Selector1/4 -> JaCycCollisonAssessInfo/4(dx)  "<dx>"
  SteerWheelAngle_Deg/1 -> TargetSelectCalc/4(SteerWheelAngle_Deg)
  Bus Selector/1 -> TargetSelectCalc/3(subjVel)  "<subjVel>"
  JaCycPrefillModSts/1 -> TargetSelectCalc/5(JaCycPrefillModSts)
  Bus Selector1/3 -> JaCycCollisonAssessInfo/3(vy)  "<vy>"
  JaCycCollisonAssessInfo/1(JaCycCollisonAssessInfo) -> Bus Creator/2  "JACycCollisonAssessInfo"
  Bus Selector2/2 -> JaCycCollisonAssessInfo/9(subjVel)  "<subjVel>"
  subjInfo/1 -> JaCycObjCalcInfoCalc/2(subjInfo), Bus Selector2/1, Bus Selector/1
  Bus Selector2/1 -> JaCycCollisonAssessInfo/8(subjYawRate)  "<subjYawRate>"
  Bus Creator/1 -> JaCycTgtCalcInfo/1

== system TosAeb/FcwAebJa_Step_sys/ALG_AEBSystem/ALG_AEBController/AEB_LatObjInfoCalcApp/LatCycObjCalc ==
  latCycObjInfo : Inport  OutDataTypeStr=Bus: BusAEBLatObj  PortDimensions=32  BusOutputAsStruct=on
  subjInfo : Inport  Port=2  OutDataTypeStr=Bus: BusSubjInfo
  Bus Creator : BusCreator  Inputs=2  OutDataTypeStr=Bus: BusLatCycTgtCalcInfo  NonVirtualBus=on
      settings: InheritFromInputs=off
  Bus Selector : BusSelector  OutputSignals=latCycObjRawInfo.ObjectID,CollisionRiskProb
  Bus Selector1 : BusSelector  OutputSignals=subjLongAccel,subjVel
  LatCycCollisonAssessInfoCalc : Subsystem
  LatCycObjCalc : Subsystem  TreatAsAtomicUnit=on
  TargetSelectCalc : Subsystem
  latCycTgtCalcInfo : Outport  OutDataTypeStr=Bus: BusLatCycTgtCalcInfo
  latCycCalcInfo : Outport  Port=2  OutDataTypeStr=Bus: BusLatCycObjCalcInfo
  wires:
  subjInfo/1 -> LatCycObjCalc/2(subjInfo), Bus Selector1/1  "subjInfo"
  LatCycCollisonAssessInfoCalc/1(latCycCollisonAssessInfo) -> Bus Creator/2  "latCycCollisonAssessInfo"
  LatCycObjCalc/1(latCycCalcInfo) -> latCycCalcInfo/1, TargetSelectCalc/1(latCycCalcInfo)  "AEB_latCycCalcInfo"
  TargetSelectCalc/1(latCycObjCalcInfo) -> Bus Selector/1, Bus Creator/1  "latCycObjCalcInfo"
  Bus Selector/2 -> LatCycCollisonAssessInfoCalc/2(cycColliisonRiskProb)  "<CollisionRiskProb>"
  Bus Selector/1 -> LatCycCollisonAssessInfoCalc/1(ObjectID)  "<ObjectID>"
  Bus Selector1/1 -> LatCycCollisonAssessInfoCalc/3(subLongAccel)  "<subjLongAccel>"
  Bus Selector1/2 -> LatCycCollisonAssessInfoCalc/4(subjVel)  "<subjVel>"
  latCycObjInfo/1 -> LatCycObjCalc/1(latCycObjInfo)
  Bus Creator/1 -> latCycTgtCalcInfo/1
  LatCycObjCalc/2(ObjPreSelectCond) -> TargetSelectCalc/2(ObjPreSelectCond)  "AEB_latCycObjPreSelectCond"

== system TosAeb/FcwAebJa_Step_sys/ALG_AEBSystem/ALG_AEBController/AEB_LatObjInfoCalcApp/LatPedObjCalc ==
  latPedObjInfo : Inport  OutDataTypeStr=Bus: BusAEBLatObj  PortDimensions=32  BusOutputAsStruct=on
  subjInfo : Inport  Port=2  OutDataTypeStr=Bus: BusSubjInfo
  FcwHMISetting : Inport  Port=3  OutDataTypeStr=Enum: FCWSnvty
  Bus Creator : BusCreator  Inputs=2  OutDataTypeStr=Bus: BusLatPedTgtCalcInfo  NonVirtualBus=on
      settings: InheritFromInputs=off
  Bus Selector : BusSelector  OutputSignals=subjVel,subjLongAccel
  Bus Selector1 : BusSelector  OutputSignals=latPedObjRawInfo.ObjectID,CollisionRiskProb,latPedObjAbsInfo.dxFrontBumper,latPedObjRawInfo.vx
  LatPedCollisonAssessInfo : Subsystem  TreatAsAtomicUnit=on
  LatPedObjCalc : Subsystem  TreatAsAtomicUnit=on
  Selector : Selector  InputPortWidth=10  IndexOptions=Index vector (dialog)  Indices=1  OutputSizes=1
  Subsystem : Subsystem
  TargetSelectCalc : Subsystem  TreatAsAtomicUnit=on
  Terminator : Terminator
  Terminator1 : Terminator
  latPedTgtCalcInfo : Outport  OutDataTypeStr=Bus: BusLatPedTgtCalcInfo
  latPedCalcInfo : Outport  Port=2  OutDataTypeStr=Bus: BusLatPedObjCalcInfo
  wires:
  Bus Selector1/2 -> LatPedCollisonAssessInfo/2(pedColliisonRisk)  "<CollisionRiskProb>"
  FcwHMISetting/1 -> LatPedCollisonAssessInfo/7(FcwHMISetting)
  Bus Selector1/1 -> LatPedCollisonAssessInfo/1(ObjectID)  "<ObjectID>"
  TargetSelectCalc/1(latPedObjCalcInfo) -> Bus Selector1/1, Bus Creator/1  "latPedTgtObj"
  Bus Selector1/3 -> LatPedCollisonAssessInfo/3(dxFrontBumper)  "<dxFrontBumper>"
  LatPedObjCalc/1(latPedCalcInfo) -> latPedCalcInfo/1, TargetSelectCalc/1(latPedCalcInfo)  "AEB_latPedCalcInfo"
  LatPedCollisonAssessInfo/1(latPedCollisonAssessInfo) -> Bus Creator/2  "latPedCollisonAssessInfo"
  Bus Creator/1 -> latPedTgtCalcInfo/1
  Bus Selector/2 -> LatPedCollisonAssessInfo/6(subLongAccel)  "<subjLongAccel>"
  subjInfo/1 -> Subsystem/2(subjInfo), Bus Selector/1, LatPedObjCalc/2(subjInfo)
  Bus Selector/1 -> LatPedCollisonAssessInfo/5(subjVel)  "<subjVel>"
  latPedObjInfo/1 -> Selector/1, LatPedObjCalc/1(latPedObjInfo)
  Bus Selector1/4 -> LatPedCollisonAssessInfo/4(vx)  "<vx>"
  LatPedObjCalc/2(ObjPreSelectCond) -> TargetSelectCalc/2(ObjPreSelectCond)  "AEB_latPedObjPreSelectCond"
  Selector/1 -> Subsystem/1(latPedObjInfo)
  Subsystem/1(latPedCalcInfo) -> Terminator/1
  Subsystem/2(ObjPreSelectCond) -> Terminator1/1

== system TosAeb/FcwAebJa_Step_sys/ALG_AEBSystem/ALG_AEBController/AEB_LongTgtInfoCalc/LongObjCalcInfoCalc ==
  longObjInfo : Inport  OutDataTypeStr=Bus: BusAEBLongObj
  subjInfo : Inport  Port=2  OutDataTypeStr=Bus: BusSubjInfo
  ACCState : Inport  Port=3  OutDataTypeStr=Enum: ACCModSts
  vehCanInfo : Inport  Port=4  OutDataTypeStr=Bus: BusVehCanInfo
  FcwHMISetting : Inport  Port=5  OutDataTypeStr=Enum: FCWSnvty
  Action Port : ActionPort  ActionPortLabel=else
  AEBS_LongCollisionAssesApp : Subsystem  TreatAsAtomicUnit=on
  Bus Creator : BusCreator  Inputs=4
  Bus Creator1 : BusCreator  Inputs=5  OutDataTypeStr=Bus: BusLongObjCalcInfo  NonVirtualBus=on
      settings: InheritFromInputs=off
  Bus Selector : BusSelector  OutputSignals=dx,vx,ax,ObjectID,ObjectTypeCameraConfirmed,ObjectType,FR_ObjectID,FR_Dx,FR_Dy,FR_Vx,FC_ObjectID,FC_Dx,FC_Dy,FC_Vx
  Bus Selector1 : BusSelector  OutputSignals=subjVel,subjLongAccel
  Bus Selector2 : BusSelector  OutputSignals=ObjectWidth,ObjectTypeCameraConfirmed,ObjectMovingState,dy,vx,vy
  Bus Selector3 : BusSelector  OutputSignals=subjLatAccel,subjYawRate,subjVel,subjCurvTraj
  Bus Selector6 : BusSelector  OutputSignals=ObjectWidth,ObjectTypeCameraConfirmed,ObjectMovingState,dyEgo2Center,dyObject2Center
  ObjAbsInfoTrans : Subsystem  TreatAsAtomicUnit=on
  OverlapCalculation : Subsystem  TreatAsAtomicUnit=on
  ProbEscapeCalcu : Subsystem  TreatAsAtomicUnit=on
  longObjCalcInfo : Outport  OutDataTypeStr=Bus: BusLongObjCalcInfo
  wires:
  subjInfo/1 -> Bus Selector1/1, AEBS_LongCollisionAssesApp/6(subjInfo), [subjInfo]
  Bus Selector1/2 -> ObjAbsInfoTrans/16(subjLongAccel)  "<subjLongAccel>"
  Bus Selector2/5 -> ProbEscapeCalcu/5(vx)  "<vx>"
  [subjInfo] -> Bus Selector3/1
  ACCState/1 -> AEBS_LongCollisionAssesApp/1(ACCState)
  Bus Selector/3 -> ObjAbsInfoTrans/3(ax)  "<ax>"
  ObjAbsInfoTrans/2(vxAbsObj) -> Bus Creator/2  "vxAbsObj"
  Bus Selector2/4 -> ProbEscapeCalcu/4(dy)  "<dy>"
  AEBS_LongCollisionAssesApp/1(LongCollisionAssess) -> Bus Creator1/3  "longCollisionAssess"
  Bus Creator/1 -> [longObjAbsInfo], AEBS_LongCollisionAssesApp/4(longObjAbsInfo)
  Bus Selector/1 -> ObjAbsInfoTrans/1(dx)  "<dx>"
  ObjAbsInfoTrans/3(axAbsObj) -> Bus Creator/3  "axAbsObj"
  Bus Selector1/1 -> ObjAbsInfoTrans/15(subjVel)  "<subjVel>"
  Bus Selector3/2 -> ProbEscapeCalcu/9(subjYawRate)  "<subjYawRate>"
  vehCanInfo/1 -> AEBS_LongCollisionAssesApp/5(vehCanInfo)
  Bus Selector/2 -> ObjAbsInfoTrans/2(vx)  "<vx>"
  ObjAbsInfoTrans/1(dxFrontBumper) -> [dxFrontBumper], Bus Creator/1  "dxFrontBumper"
  [dxFrontBumper] -> ProbEscapeCalcu/7(dxFrontBumper)  "dxFrontBumper"
  Bus Creator1/1 -> longObjCalcInfo/1
  OverlapCalculation/1(overlapRatio) -> Bus Creator1/5  "overlapRatio"
  Bus Selector6/2 -> OverlapCalculation/2(ObjectTypeCameraConfirmed)  "<ObjectTypeCameraConfirmed>"
  Bus Selector2/1 -> ProbEscapeCalcu/1(ObjectWidth)  "<ObjectWidth>"
  FcwHMISetting/1 -> AEBS_LongCollisionAssesApp/2(FcwHMISetting)
  Bus Selector3/4 -> ProbEscapeCalcu/11(subjCurvTraj)  "<subjCurvTraj>"
  Bus Selector6/4 -> OverlapCalculation/4(dyEgo2Center)  "<dyEgo2Center>"
  Bus Selector6/5 -> OverlapCalculation/5(dyObject2Center)  "<dyObject2Center>"
  [longObjAbsInfo] -> Bus Creator1/2  "longObjAbsInfo"
  ProbEscapeCalcu/1(probEscape) -> Bus Creator1/4  "probEscape"
  Bus Selector3/3 -> ProbEscapeCalcu/10(subjVel)  "<subjVel>"
  Bus Selector6/1 -> OverlapCalculation/1(ObjectWidth)  "<ObjectWidth>"
  longObjInfo/1 -> AEBS_LongCollisionAssesApp/3(longObjInfo), Bus Creator1/1, Bus Selector6/1, Bus Selector2/1, Bus Selector/1  "longObjInfo"
  Bus Selector6/3 -> OverlapCalculation/3(ObjectMovingState)  "<ObjectMovingState>"
  Bus Selector2/2 -> ProbEscapeCalcu/2(ObjectTypeCameraConfirmed)  "<ObjectTypeCameraConfirmed>"
  Bus Selector2/6 -> ProbEscapeCalcu/6(vy)  "<vy>"
  Bus Selector3/1 -> ProbEscapeCalcu/8(subjLatAccel)  "<subjLatAccel>"
  Bus Selector2/3 -> ProbEscapeCalcu/3(ObjectMovingState)  "<ObjectMovingState>"
  Bus Selector/4 -> ObjAbsInfoTrans/4(ObjectID)  "<ObjectID>"
  Bus Selector/5 -> ObjAbsInfoTrans/5(ObjectTypeCameraConfirmed)  "<ObjectTypeCameraConfirmed>"
  Bus Selector/7 -> ObjAbsInfoTrans/7(FR_ObjectID)  "<FR_ObjectID>"
  Bus Selector/8 -> ObjAbsInfoTrans/8(FR_Dx)  "<FR_Dx>"
  Bus Selector/9 -> ObjAbsInfoTrans/9(FR_Dy)  "<FR_Dy>"
  Bus Selector/11 -> ObjAbsInfoTrans/11(FC_ObjectID)  "<FC_ObjectID>"
  Bus Selector/12 -> ObjAbsInfoTrans/12(FC_Dx)  "<FC_Dx>"
  Bus Selector/13 -> ObjAbsInfoTrans/13(FC_Dy)  "<FC_Dy>"
  ObjAbsInfoTrans/4(ObjFusQuality) -> Bus Creator/4  "ObjFusQuality"
  Bus Selector/14 -> ObjAbsInfoTrans/14(FC_Vx)  "<FC_Vx>"
  Bus Selector/10 -> ObjAbsInfoTrans/10(FR_Vx)  "<FR_Vx>"
  Bus Selector/6 -> ObjAbsInfoTrans/6(ObjectType)  "<ObjectType>"

== system TosAeb/FcwAebJa_Step_sys/ALG_AEBSystem/ALG_AEBController/AEB_LongTgtInfoCalc/NoTarget ==
  Action Port : ActionPort  ActionPortLabel=if(u1)
  longObjCalcInfoDefaultParams : Constant  Value=longObjCalcInfoDefaultParams  OutDataTypeStr=Bus: BusLongObjCalcInfo
  longObjCalcInfo : Outport  OutDataTypeStr=Bus: BusLongObjCalcInfo
  wires:
  longObjCalcInfoDefaultParams/1 -> longObjCalcInfo/1

== system TosAeb/FcwAebJa_Step_sys/ALG_AEBSystem/ALG_AEBController/AEB_LongTgtInfoCalc/ObjISInvalid ==
  ObjectID : Inport
  Compare To Constant : simulink/Logic and Bit Operations/Compare To Constant  relop===  const=CNobjInvalidID  OutDataTypeStr=boolean
  ObjIsInvalidFlg : Outport
  wires:
  ObjectID/1 -> Compare To Constant/1
  Compare To Constant/1 -> ObjIsInvalidFlg/1
